simulink library "Garbage"  (R2023a)
settings: Solver=ode45  StartTime=0.0  StopTime=10.0  RelTol=1e-3  EnableMultiTasking=on
undefined symbols (not defined in the file or in its folder): c, ending_column_index, ending_row_index, framebuffer_16x1, framebuffer_2x2, framebuffer_4x4, framebuffer_8x8, HEIGHT, hot, POS_X, POS_Y, starting_column_index, starting_row_index, WIDTH
libraries: Memory_Blocks_Lib x459, simulink x235, simulink_extras x132, dspmtrx3 x116, Bit_Multiplexers_Lib x67, Bit_Demultiplexers_Lib x49, Byte_Multiplexers_Lib x40, Miscellaneous x38, Computer_Library x17, Decoders_Lib x14, commutil2 x12, Arithmetic_Operations_Lib x8
(part 26 of 26)

== system Garbage/Text_Display_1x16/Character_Table/Characters(Q..ARROW_UP)/Char_PLUS ==
  Byte_01 : Constant  Value=c.char(2, c.PLUS)  OutDataTypeStr=uint8
  Byte_02 : Constant  Value=c.char(3, c.PLUS)  OutDataTypeStr=uint8
  Byte_03 : Constant  Value=c.char(4, c.PLUS)  OutDataTypeStr=uint8
  Byte_04 : Constant  Value=c.char(5, c.PLUS)  OutDataTypeStr=uint8
  Byte_05 : Constant  Value=c.char(6, c.PLUS)  OutDataTypeStr=uint8
  Byte_06 : Constant  Value=c.char(7, c.PLUS)  OutDataTypeStr=uint8
  Byte_07 : Constant  Value=c.char(8, c.PLUS)  OutDataTypeStr=uint8
  Byte_1 : Constant  Value=c.char(1, c.PLUS)  OutDataTypeStr=uint8
  Row_7(1B) : Outport
  Row_6(1B) : Outport  Port=2
  Row_5(1B) : Outport  Port=3
  Row_4(1B) : Outport  Port=4
  Row_3(1B) : Outport  Port=5
  Row_2(1B) : Outport  Port=6
  Row_1(1B) : Outport  Port=7
  Row_0(1B) : Outport  Port=8
  wires:
  Byte_02/1 -> Row_5(1B)/1
  Byte_1/1 -> Row_7(1B)/1
  Byte_06/1 -> Row_1(1B)/1
  Byte_03/1 -> Row_4(1B)/1
  Byte_07/1 -> Row_0(1B)/1
  Byte_01/1 -> Row_6(1B)/1
  Byte_04/1 -> Row_3(1B)/1
  Byte_05/1 -> Row_2(1B)/1

== system Garbage/Text_Display_1x16/Character_Table/Characters(Q..ARROW_UP)/Char_Q ==
  Byte_01 : Constant  Value=c.char(2, c.Q)  OutDataTypeStr=uint8
  Byte_02 : Constant  Value=c.char(3, c.Q)  OutDataTypeStr=uint8
  Byte_03 : Constant  Value=c.char(4, c.Q)  OutDataTypeStr=uint8
  Byte_04 : Constant  Value=c.char(5, c.Q)  OutDataTypeStr=uint8
  Byte_05 : Constant  Value=c.char(6, c.Q)  OutDataTypeStr=uint8
  Byte_06 : Constant  Value=c.char(7, c.Q)  OutDataTypeStr=uint8
  Byte_07 : Constant  Value=c.char(8, c.Q)  OutDataTypeStr=uint8
  Byte_1 : Constant  Value=c.char(1, c.Q)  OutDataTypeStr=uint8
  Row_7(1B) : Outport
  Row_6(1B) : Outport  Port=2
  Row_5(1B) : Outport  Port=3
  Row_4(1B) : Outport  Port=4
  Row_3(1B) : Outport  Port=5
  Row_2(1B) : Outport  Port=6
  Row_1(1B) : Outport  Port=7
  Row_0(1B) : Outport  Port=8
  wires:
  Byte_02/1 -> Row_5(1B)/1
  Byte_1/1 -> Row_7(1B)/1
  Byte_06/1 -> Row_1(1B)/1
  Byte_03/1 -> Row_4(1B)/1
  Byte_07/1 -> Row_0(1B)/1
  Byte_01/1 -> Row_6(1B)/1
  Byte_04/1 -> Row_3(1B)/1
  Byte_05/1 -> Row_2(1B)/1

== system Garbage/Text_Display_1x16/Character_Table/Characters(Q..ARROW_UP)/Char_SEMICOLON ==
  Byte_01 : Constant  Value=c.char(2, c.SEMICOLON)  OutDataTypeStr=uint8
  Byte_02 : Constant  Value=c.char(3, c.SEMICOLON)  OutDataTypeStr=uint8
  Byte_03 : Constant  Value=c.char(4, c.SEMICOLON)  OutDataTypeStr=uint8
  Byte_04 : Constant  Value=c.char(5, c.SEMICOLON)  OutDataTypeStr=uint8
  Byte_05 : Constant  Value=c.char(6, c.SEMICOLON)  OutDataTypeStr=uint8
  Byte_06 : Constant  Value=c.char(7, c.SEMICOLON)  OutDataTypeStr=uint8
  Byte_07 : Constant  Value=c.char(8, c.SEMICOLON)  OutDataTypeStr=uint8
  Byte_1 : Constant  Value=c.char(1, c.SEMICOLON)  OutDataTypeStr=uint8
  Row_7(1B) : Outport
  Row_6(1B) : Outport  Port=2
  Row_5(1B) : Outport  Port=3
  Row_4(1B) : Outport  Port=4
  Row_3(1B) : Outport  Port=5
  Row_2(1B) : Outport  Port=6
  Row_1(1B) : Outport  Port=7
  Row_0(1B) : Outport  Port=8
  wires:
  Byte_02/1 -> Row_5(1B)/1
  Byte_1/1 -> Row_7(1B)/1
  Byte_06/1 -> Row_1(1B)/1
  Byte_03/1 -> Row_4(1B)/1
  Byte_07/1 -> Row_0(1B)/1
  Byte_01/1 -> Row_6(1B)/1
  Byte_04/1 -> Row_3(1B)/1
  Byte_05/1 -> Row_2(1B)/1

== system Garbage/Text_Display_1x16/Character_Table/Characters(Q..ARROW_UP)/Char_SLASH ==
  Byte_01 : Constant  Value=c.char(2, c.SLASH)  OutDataTypeStr=uint8
  Byte_02 : Constant  Value=c.char(3, c.SLASH)  OutDataTypeStr=uint8
  Byte_03 : Constant  Value=c.char(4, c.SLASH)  OutDataTypeStr=uint8
  Byte_04 : Constant  Value=c.char(5, c.SLASH)  OutDataTypeStr=uint8
  Byte_05 : Constant  Value=c.char(6, c.SLASH)  OutDataTypeStr=uint8
  Byte_06 : Constant  Value=c.char(7, c.SLASH)  OutDataTypeStr=uint8
  Byte_07 : Constant  Value=c.char(8, c.SLASH)  OutDataTypeStr=uint8
  Byte_1 : Constant  Value=c.char(1, c.SLASH)  OutDataTypeStr=uint8
  Row_7(1B) : Outport
  Row_6(1B) : Outport  Port=2
  Row_5(1B) : Outport  Port=3
  Row_4(1B) : Outport  Port=4
  Row_3(1B) : Outport  Port=5
  Row_2(1B) : Outport  Port=6
  Row_1(1B) : Outport  Port=7
  Row_0(1B) : Outport  Port=8
  wires:
  Byte_02/1 -> Row_5(1B)/1
  Byte_1/1 -> Row_7(1B)/1
  Byte_06/1 -> Row_1(1B)/1
  Byte_03/1 -> Row_4(1B)/1
  Byte_07/1 -> Row_0(1B)/1
  Byte_01/1 -> Row_6(1B)/1
  Byte_04/1 -> Row_3(1B)/1
  Byte_05/1 -> Row_2(1B)/1

== system Garbage/Text_Display_1x16/Character_Table/Characters(Q..ARROW_UP)/Char_X ==
  Byte_01 : Constant  Value=c.char(2, c.X)  OutDataTypeStr=uint8
  Byte_02 : Constant  Value=c.char(3, c.X)  OutDataTypeStr=uint8
  Byte_03 : Constant  Value=c.char(4, c.X)  OutDataTypeStr=uint8
  Byte_04 : Constant  Value=c.char(5, c.X)  OutDataTypeStr=uint8
  Byte_05 : Constant  Value=c.char(6, c.X)  OutDataTypeStr=uint8
  Byte_06 : Constant  Value=c.char(7, c.X)  OutDataTypeStr=uint8
  Byte_07 : Constant  Value=c.char(8, c.X)  OutDataTypeStr=uint8
  Byte_1 : Constant  Value=c.char(1, c.X)  OutDataTypeStr=uint8
  Row_7(1B) : Outport
  Row_6(1B) : Outport  Port=2
  Row_5(1B) : Outport  Port=3
  Row_4(1B) : Outport  Port=4
  Row_3(1B) : Outport  Port=5
  Row_2(1B) : Outport  Port=6
  Row_1(1B) : Outport  Port=7
  Row_0(1B) : Outport  Port=8
  wires:
  Byte_02/1 -> Row_5(1B)/1
  Byte_1/1 -> Row_7(1B)/1
  Byte_06/1 -> Row_1(1B)/1
  Byte_03/1 -> Row_4(1B)/1
  Byte_07/1 -> Row_0(1B)/1
  Byte_01/1 -> Row_6(1B)/1
  Byte_04/1 -> Row_3(1B)/1
  Byte_05/1 -> Row_2(1B)/1

== system Garbage/Text_Display_1x16/Character_Table/Characters(Q..ARROW_UP)/Char_Y ==
  Byte_01 : Constant  Value=c.char(2, c.Y)  OutDataTypeStr=uint8
  Byte_02 : Constant  Value=c.char(3, c.Y)  OutDataTypeStr=uint8
  Byte_03 : Constant  Value=c.char(4, c.Y)  OutDataTypeStr=uint8
  Byte_04 : Constant  Value=c.char(5, c.Y)  OutDataTypeStr=uint8
  Byte_05 : Constant  Value=c.char(6, c.Y)  OutDataTypeStr=uint8
  Byte_06 : Constant  Value=c.char(7, c.Y)  OutDataTypeStr=uint8
  Byte_07 : Constant  Value=c.char(8, c.Y)  OutDataTypeStr=uint8
  Byte_1 : Constant  Value=c.char(1, c.Y)  OutDataTypeStr=uint8
  Row_7(1B) : Outport
  Row_6(1B) : Outport  Port=2
  Row_5(1B) : Outport  Port=3
  Row_4(1B) : Outport  Port=4
  Row_3(1B) : Outport  Port=5
  Row_2(1B) : Outport  Port=6
  Row_1(1B) : Outport  Port=7
  Row_0(1B) : Outport  Port=8
  wires:
  Byte_02/1 -> Row_5(1B)/1
  Byte_1/1 -> Row_7(1B)/1
  Byte_06/1 -> Row_1(1B)/1
  Byte_03/1 -> Row_4(1B)/1
  Byte_07/1 -> Row_0(1B)/1
  Byte_01/1 -> Row_6(1B)/1
  Byte_04/1 -> Row_3(1B)/1
  Byte_05/1 -> Row_2(1B)/1

== system Garbage/Text_Display_1x16/Character_Table/Characters(Q..ARROW_UP)/Char_Z ==
  Byte_01 : Constant  Value=c.char(2, c.Z)  OutDataTypeStr=uint8
  Byte_02 : Constant  Value=c.char(3, c.Z)  OutDataTypeStr=uint8
  Byte_03 : Constant  Value=c.char(4, c.Z)  OutDataTypeStr=uint8
  Byte_04 : Constant  Value=c.char(5, c.Z)  OutDataTypeStr=uint8
  Byte_05 : Constant  Value=c.char(6, c.Z)  OutDataTypeStr=uint8
  Byte_06 : Constant  Value=c.char(7, c.Z)  OutDataTypeStr=uint8
  Byte_07 : Constant  Value=c.char(8, c.Z)  OutDataTypeStr=uint8
  Byte_1 : Constant  Value=c.char(1, c.Z)  OutDataTypeStr=uint8
  Row_7(1B) : Outport
  Row_6(1B) : Outport  Port=2
  Row_5(1B) : Outport  Port=3
  Row_4(1B) : Outport  Port=4
  Row_3(1B) : Outport  Port=5
  Row_2(1B) : Outport  Port=6
  Row_1(1B) : Outport  Port=7
  Row_0(1B) : Outport  Port=8
  wires:
  Byte_02/1 -> Row_5(1B)/1
  Byte_1/1 -> Row_7(1B)/1
  Byte_06/1 -> Row_1(1B)/1
  Byte_03/1 -> Row_4(1B)/1
  Byte_07/1 -> Row_0(1B)/1
  Byte_01/1 -> Row_6(1B)/1
  Byte_04/1 -> Row_3(1B)/1
  Byte_05/1 -> Row_2(1B)/1

== matlab function Garbage/Screen_40x25__/MATLAB Function ==
  data: Framebuffer output uint8 size=[8*25 8*40]; CharTable input uint8 size=[8 8 256]; VideoMemory input uint8 size=1024
function Framebuffer = Video(CharTable, VideoMemory)
    VIDEO_MEM_SIZE = 1000;
    MAX_X = 40;
    MAX_Y = 25;
    CHAR_SIZE = 8;
    Framebuffer = zeros(MAX_Y*CHAR_SIZE, MAX_X*CHAR_SIZE, 'uint8');

    for (i = 1 : VIDEO_MEM_SIZE)
        y = idivide(uint16(i) - 1, uint16(MAX_X)) + 1;
        x = mod(uint16(i) - 1, uint16(MAX_X)) + 1;

        if (0 == VideoMemory(i))
            % Interpret as a space/blank character.
            ordNum = uint8(32);
        else
            ordNum = VideoMemory(i);
        end
        
        Framebuffer((CHAR_SIZE * (y - 1) + 1) : (CHAR_SIZE * (y - 1) + CHAR_SIZE),  ...
                    (CHAR_SIZE * (x - 1) + 1) : (CHAR_SIZE * (x - 1) + CHAR_SIZE))  ...
            = CharTable(:, :, ordNum);
    end
end
